simulink model "trustai_simulation_cl"  (R2022b)
settings: Solver=FixedStepAuto  StartTime=0.0  StopTime=45  FixedStep=0.05  RelTol=1e-3
model workspace (MAT data in the model file):
  Logfile_Data_Object : robotics.ros.BagSelection (value not decoded)
  Logfile_Path = '/Users/sprinkle/work/teaching/cs3891-2023F/src/docker/mytest.bag'
  Logfile_Playback_Duration = 200
  Logfile_Playback_Offset = 100
libraries: simulink x1
settings Model: Creator=sprinkle  LastModifiedBy=sprinkle
settings SolverCC: AlgebraicLoopSolver=TrustRegion
settings OptimizationCC: EnhancedBackFolding=on  PassReuseOutputArgsAs=Individual arguments  ZeroExternalMemoryAtStartup=off  ZeroInternalMemoryAtStartup=off  MaxStackSize=64  OptimizeBlockOrder=speed  DifferentSizesBufferReuse=on  OptimizationCustomize=off  EfficientTunableParamExpr=on
settings DebuggingCC: ParameterTunabilityLossMsg=error  UnconnectedInputMsg=warning  UnconnectedOutputMsg=warning  UnconnectedLineMsg=warning
settings HardwareCC: ProdBitPerPointer=32  ProdBitPerSizeT=32  ProdBitPerPtrDiffT=32  ProdLargestAtomicFloat=None  ProdIntDivRoundTo=Undefined  ProdEndianess=Unspecified  ProdWordSize=32  ProdHWDeviceType=Generic->Unspecified (assume 32-bit Generic)
settings ModelReferenceCC: EnableRefExpFcnMdlSchedulingChecks=on
settings SFSimCC: MATLABDynamicMemAlloc=off
settings RTWCC: Description=Embedded Coder  SystemTargetFile=ert.tlc  HardwareBoard=Robot Operating System (ROS)  TLCOptions="-aInlineSetEventsForThisBaseRateFcn=TLC_FALSE -aSuppressMultiTaskScheduler=TLC_FALSE  -aRateBasedStepFcn=0"  TemplateMakefile=ert_default_tmf  Toolchain=Catkin  TargetLang=C++
settings CodeAppCC: ForceParamTrailComments=on  IgnoreCustomStorageClasses=off  OperatorAnnotations=on  SimulinkDataObjDesc=on  SFDataObjDesc=on  InsertBlockDesc=on
settings ERTTargetCC: SupportContinuousTime=on

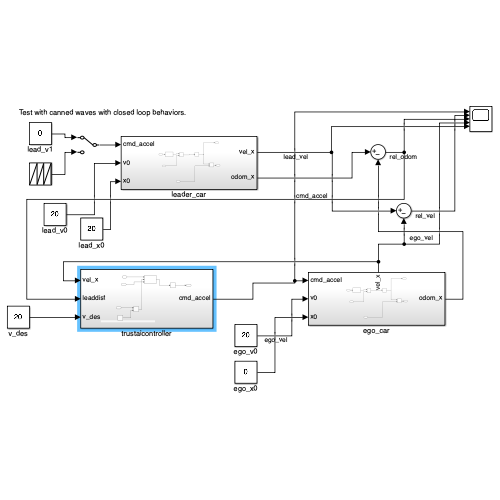
[thumbnail: Simulink's stored preview of the top-level diagram]
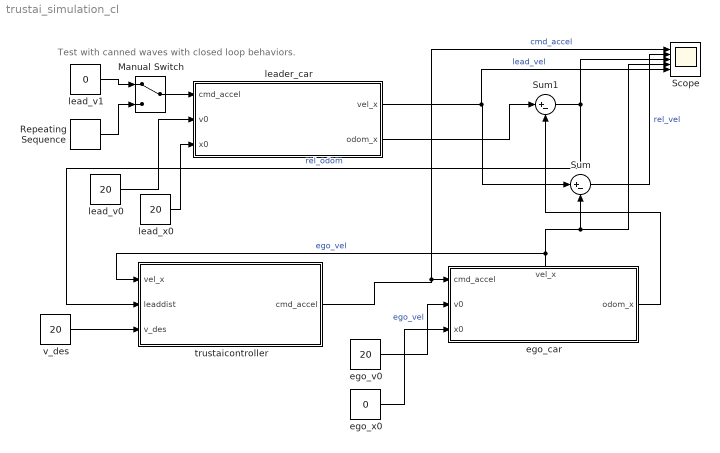
== system trustai_simulation_cl ==
  note: Test with canned waves with closed loop behaviors.
  Manual Switch : ManualSwitch
  Repeating Sequence : simulink/Sources/Repeating Sequence  rep_seq_t=[0 20]  rep_seq_y=[-2 2]
  Scope : Scope  NumInputPorts=5
  Sum : Sum  Inputs=|+-
  Sum1 : Sum  Inputs=|+-
  ego_car : Subsystem
      settings: PortSchema="{\"entries\":[{\"content\":{\"sides\":[{\"content\":{\"connectorIds\":[\"In1\",\"In2\",\"In3\"]},\"type\":\"ConnectorPlacement.EquallySpacedRectSide\",\"uuid\":\"81953a61-2087-4640-8924-dd2553d262e4\"},{\"content\":{\"connectorIds\":[\"Out1\"],\"side\":\"RIGHT\"},\"type\":\"ConnectorPlacement.EquallySpacedRectSide\",\"uuid\":\"3438fc64-880d-4339-a3b8-3c07203bfc0a\"},{\"content\":{\"connectorIds\":[\"Out2\"],\"side\":\"TOP\"},\"type\":\"ConnectorPlacement.EquallySpacedRectSide\",\"uuid\":\"5fb53f00-e2cd-4409-87c6-d9d884618a68\"}]},\"type\":\"ConnectorPlacement.EquallySpacedConnector\",\"uuid\":\"e0e50f07-b074-4548-b861-e4b426313971\"}],\"packageUris\":[\"http://schema.mathworks.com/mf0/Connector/R2020b\"],\"version\":\"1.0\"}"
  ego_v0 : Constant  Value=20
  ego_x0 : Constant  Value=0
  lead_v0 : Constant  Value=20
  lead_v1 : Constant  Value=0
  lead_x0 : Constant  Value=20
  leader_car : Subsystem
  trustaicontroller : Subsystem
  v_des : Constant  Value=20
  wires:
  Manual Switch/1 -> leader_car/1(cmd_accel)
  lead_v1/1 -> Manual Switch/1
  Repeating Sequence/1 -> Manual Switch/2
  leader_car/1(vel_x) -> Scope/5, Sum/1  "lead_vel"
  ego_car/2(vel_x) -> trustaicontroller/1(vel_x), Scope/4, Sum/2  "ego_vel"
  lead_v0/1 -> leader_car/2(v0)
  lead_x0/1 -> leader_car/3(x0)
  ego_v0/1 -> ego_car/2(v0)  "ego_vel"
  ego_x0/1 -> ego_car/3(x0)
  leader_car/2(odom_x) -> Sum1/1
  ego_car/1(odom_x) -> Sum1/2
  Sum1/1 -> Scope/3, trustaicontroller/2(leaddist)  "rel_odom"
  trustaicontroller/1(cmd_accel) -> Scope/1, ego_car/1(cmd_accel)  "cmd_accel"
  Sum/1 -> Scope/2  "rel_vel"
  v_des/1 -> trustaicontroller/3(v_des)

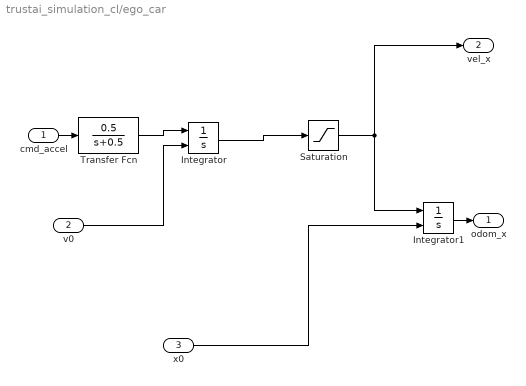
== system trustai_simulation_cl/ego_car ==
  cmd_accel : Inport
  v0 : Inport  Port=2
  x0 : Inport  Port=3
  Integrator : Integrator  InitialConditionSource=external
  Integrator1 : Integrator  InitialConditionSource=external
  Saturation : Saturate  UpperLimit=80  LowerLimit=0
  Transfer Fcn : TransferFcn  Numerator=[0.5]  Denominator=[1 0.5]
  odom_x : Outport
  vel_x : Outport  Port=2
  wires:
  v0/1 -> Integrator/2
  cmd_accel/1 -> Transfer Fcn/1
  Transfer Fcn/1 -> Integrator/1
  Integrator1/1 -> odom_x/1
  Integrator/1 -> Saturation/1
  x0/1 -> Integrator1/2
  Saturation/1 -> vel_x/1, Integrator1/1

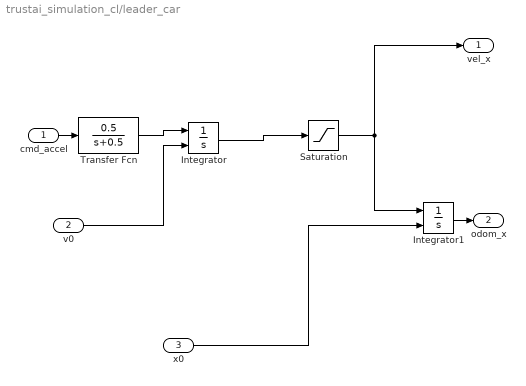
== system trustai_simulation_cl/leader_car ==
  cmd_accel : Inport
  v0 : Inport  Port=2
  x0 : Inport  Port=3
  Integrator : Integrator  InitialConditionSource=external
  Integrator1 : Integrator  InitialConditionSource=external
  Saturation : Saturate  UpperLimit=80  LowerLimit=0
  Transfer Fcn : TransferFcn  Numerator=[0.5]  Denominator=[1 0.5]
  vel_x : Outport
  odom_x : Outport  Port=2
  wires:
  v0/1 -> Integrator/2
  cmd_accel/1 -> Transfer Fcn/1
  Transfer Fcn/1 -> Integrator/1
  Integrator1/1 -> odom_x/1
  Integrator/1 -> Saturation/1
  x0/1 -> Integrator1/2
  Saturation/1 -> vel_x/1, Integrator1/1

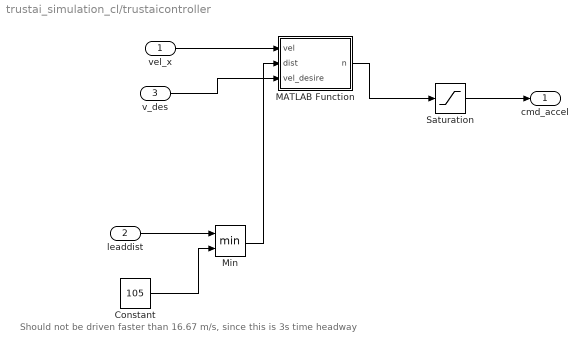
== system trustai_simulation_cl/trustaicontroller ==
  note: Should not be driven faster than 16.67 m/s, since this is 3s time headway
  vel_x : Inport
  leaddist : Inport  Port=2
  v_des : Inport  Port=3
  Constant : Constant  Value=105
  MATLAB Function : MATLAB Function  TreatAsAtomicUnit=on
  Min : MinMax  Inputs=2
  Saturation : Saturate
  cmd_accel : Outport
  wires:
  MATLAB Function/1(n) -> Saturation/1
  vel_x/1 -> MATLAB Function/1(vel)
  leaddist/1 -> Min/1
  Constant/1 -> Min/2
  Saturation/1 -> cmd_accel/1
  Min/1 -> MATLAB Function/2(dist)
  v_des/1 -> MATLAB Function/3(vel_desire)

== matlab function trustai_simulation_cl/trustaicontroller/MATLAB Function ==
  data: vel input; dist input; n output; vel_desire input
function n  = fcn(vel, dist, vel_desire)
    k1 = 0.1;
    k2 = 0.1;
    headway = 3;
%     vel_desire = 15;

    n = k1 * (dist - headway * vel) + k2 * (vel_desire - vel);
end
